simulink model "AcasXuClosedLoop_all_comp2017b"  (Simulink 9.0)
settings: Solver=VariableStepAuto  StartTime=0.0  StopTime=20  RelTol=1e-3
libraries: neural x14040, simulink x2
(part 11 of 534)

== system AcasXuClosedLoop_all_comp2017b/networks/Networks_m_2/AcasXu_2_2/Process Input 1 ==
  x : Inport  PortDimensions=5
      mask Display: e = 0.06;c1 = [120 230 180]/255;c2 = ([120 230 180]+0)/(256+128);c3 = ([120 230 180]+128)/(256+128);patch([0 1 1 0],[0 0 1 1],c1);patch([0 1 1 1-e 1-e 0+e],[0 0 1 1-e 0+e 0+e],c2);patch([0 0 1 1-e 0+e 0+e],[0 1 1 1-e 1-e 0+e],c3);plot([0 1 1 0 0],[0 0 1 1 0]);
  p : Outport  PortDimensions=5
      mask Display: e = 0.06;c1 = [120 230 180]/255;c2 = ([120 230 180]+0)/(256+128);c3 = ([120 230 180]+128)/(256+128);patch([0 1 1 0],[0 0 1 1],c1);patch([0 1 1 1-e 1-e 0+e],[0 0 1 1-e 0+e 0+e],c2);patch([0 0 1 1-e 0+e 0+e],[0 1 1 1-e 1-e 0+e],c3);plot([0 1 1 0 0],[0 0 1 1 0]);
  wires:
  x/1 -> p/1

== system AcasXuClosedLoop_all_comp2017b/networks/Networks_m_2/AcasXu_2_2/Process Output 1 ==
  a : Inport  PortDimensions=5
      mask Display: e = 0.06;c1 = [120 230 180]/255;c2 = ([120 230 180]+0)/(256+128);c3 = ([120 230 180]+128)/(256+128);patch([0 1 1 0],[0 0 1 1],c1);patch([0 1 1 1-e 1-e 0+e],[0 0 1 1-e 0+e 0+e],c2);patch([0 0 1 1-e 0+e 0+e],[0 1 1 1-e 1-e 0+e],c3);plot([0 1 1 0 0],[0 0 1 1 0]);
  y : Outport  PortDimensions=5
      mask Display: e = 0.06;c1 = [120 230 180]/255;c2 = ([120 230 180]+0)/(256+128);c3 = ([120 230 180]+128)/(256+128);patch([0 1 1 0],[0 0 1 1],c1);patch([0 1 1 1-e 1-e 0+e],[0 0 1 1-e 0+e 0+e],c2);patch([0 0 1 1-e 0+e 0+e],[0 1 1 1-e 1-e 0+e],c3);plot([0 1 1 0 0],[0 0 1 1 0]);
  wires:
  a/1 -> y/1

== system AcasXuClosedLoop_all_comp2017b/networks/Networks_m_2/AcasXu_3_2/Layer 1 ==
  "p{1}" : Inport  PortDimensions=5
      mask Display: e = 0.06;c1 = [120 230 180]/255;c2 = ([120 230 180]+0)/(256+128);c3 = ([120 230 180]+128)/(256+128);patch([0 1 1 0],[0 0 1 1],c1);patch([0 1 1 1-e 1-e 0+e],[0 0 1 1-e 0+e 0+e],c2);patch([0 0 1 1-e 0+e 0+e],[0 1 1 1-e 1-e 0+e],c3);plot([0 1 1 0 0],[0 0 1 1 0]);
  Delays 1 : Subsystem
      mask Display: e = 0.06;c1 = [120 150 230]/255;c2 = ([120 150 230]+0)/383;c3 = ([120 150 230]+128)/383;patch([0 1 1 0],[0 0 1 1],c1);patch([0 1 1 1-e 1-e 0+e],[0 0 1 1-e 0+e 0+e],c2);patch([0 0 1 1-e 0+e 0+e],[0 1 1 1-e 1-e 0+e],c3);plot([0 1 1 0 0],[0 0 1 1 0]);disp('0');
  "IW{1,1}" : Subsystem
      mask Display: e = 0.06;c1 = [120 150 230]/255;c2 = ([120 150 230]+0)/383;c3 = ([120 150 230]+128)/383;patch([0 1 1 0],[0 0 1 1],c1);patch([0 1 1 1-e 1-e 0+e],[0 0 1 1-e 0+e 0+e],c2);patch([0 0 1 1-e 0+e 0+e],[0 1 1 1-e 1-e 0+e],c3);plot([0 1 1 0 0],[0 0 1 1 0]);disp('W');
  "b{1}" : Constant  Value=[0.0283022999999999989861887428332920535467565059661865234375;-0.301012000000000001787014980436651967465877532958984375;0.11993800000000000294253510446651489473879337310791015625;0.2316000000000000003108624468950438313186168670654296875;-0.11227900000000000380051545789683586917817592620849609375;-0.417827000000000003954170324504957534372806549072265625;-0.65632100000000004325073632571729831397533416748046875;-0.200220000000000009077183449335279874503612518310546875;-0.388517999999999974480857645176001824438571929931640625;-0.47899300000000000210320649784989655017852783203125;-0.0209766000000000014946710535923557472415268421173095703125;-0.00976452000000000049084736275517570902593433856964111328125;-0.017845300000000001439115493440112913958728313446044921875;-0.1511429999999999995718980017045396380126476287841796875;0.10026500000000000689670542897147242911159992218017578125;-0.28527299999999999879918277656543068587779998779296875;-0.3036659999999999914876980255939997732639312744140625;-0.196284999999999987263521461500204168260097503662109375;-0.111543000000000003257838443460059352219104766845703125;-0.69079800000000002313527147634886205196380615234375;-0.021684599999999998320987515398883260786533355712890625;-0.538942000000000032144953365786932408809661865234375;0.240161000000000013354650718611082993447780609130859375;-0.469312000000000006938449814697378315031528472900390625;-0.44349899999999997657340600198949687182903289794921875;-0.661278999999999950176743368501774966716766357421875;-0.012396600000000000674216238394365063868463039398193359375;-0.1601599999999999968114394732765504159033298492431640625;-0.259077999999999974978237560208071954548358917236328125;0.0317875999999999991896260098656057380139827728271484375;0.0017260999999999999433841768592401422210969030857086181640625;-0.295569000000000026151525389650487340986728668212890625;0.151245999999999991558752299170009791851043701171875;0.07170700000000000684519108062886516563594341278076171875;0.01310560000000000023534507676004068343900144100189208984375;0.12482000000000000039523939676655572839081287384033203125;-0.486669999999999991491250739272800274193286895751953125;0.1386159999999999892228430553586804307997226715087890625;-0.4859510000000000218278728425502777099609375;-0.1790609999999999979447551368139102123677730560302734375;0.461324999999999985078602549037896096706390380859375;-0.01060919999999999918660620323862531222403049468994140625;0.45652700000000001612221467439667321741580963134765625;0.09737079999999999346815826584133901633322238922119140625;0.17900199999999999445066123371361754834651947021484375;-0.856346999999999969332975524594075977802276611328125;-0.52729499999999995818456000051810406148433685302734375;-0.69184900000000004727240821011946536600589752197265625;-0.194915000000000004920508445138693787157535552978515625;-0.642387999999999959044316710787825286388397216796875]
      mask Display: e = 0.06;c1 = [120 150 230]/255;c2 = ([120 150 230]+0)/383;c3 = ([120 150 230]+128)/383;patch([0 1 1 0],[0 0 1 1],c1);patch([0 1 1 1-e 1-e 0+e],[0 0 1 1-e 0+e 0+e],c2);patch([0 0 1 1-e 0+e 0+e],[0 1 1 1-e 1-e 0+e],c3);plot([0 1 1 0 0],[0 0 1 1 0]);disp('b');
  netsum : Sum  Inputs=2  SaturateOnIntegerOverflow=on  InputSameDT=on  OutDataTypeStr=Inherit: Same as first input
      mask Display: e = 0.06;c1 = [120 150 230]/255;c2 = ([120 150 230]+0)/383;c3 = ([120 150 230]+128)/383;patch([0 1 1 0],[0 0 1 1],c1);patch([0 1 1 1-e 1-e 0+e],[0 0 1 1-e 0+e 0+e],c2);patch([0 0 1 1-e 0+e 0+e],[0 1 1 1-e 1-e 0+e],c3);plot([0 1 1 0 0],[0 0 1 1 0]);disp('+');
  poslin : neural/Transfer Functions/poslin
      settings: SourceProductName=Deep Learning Toolbox  SourceProductBaseCode=NN
  "a{1}" : Outport  InitialOutput=[0;0;0;0;0;0;0;0;0;0;0;0;0;0;0;0;0;0;0;0;0;0;0;0;0;0;0;0;0;0;0;0;0;0;0;0;0;0;0;0;0;0;0;0;0;0;0;0;0;0]
      mask Display: e = 0.06;c1 = [120 230 180]/255;c2 = ([120 230 180]+0)/(256+128);c3 = ([120 230 180]+128)/(256+128);patch([0 1 1 0],[0 0 1 1],c1);patch([0 1 1 1-e 1-e 0+e],[0 0 1 1-e 0+e 0+e],c2);patch([0 0 1 1-e 0+e 0+e],[0 1 1 1-e 1-e 0+e],c3);plot([0 1 1 0 0],[0 0 1 1 0]);
  wires:
  poslin/1 -> "a{1}"/1
  netsum/1 -> poslin/1
  "b{1}"/1 -> netsum/2
  "IW{1,1}"/1("iz{1,1}") -> netsum/1
  Delays 1/1("pd{1,1}") -> "IW{1,1}"/1("pd{1,1}")
  "p{1}"/1 -> Delays 1/1("p{1}")

== system AcasXuClosedLoop_all_comp2017b/networks/Networks_m_2/AcasXu_3_2/Layer 2 ==
  "a{1} " : Inport  PortDimensions=50
      mask Display: e = 0.06;c1 = [120 230 180]/255;c2 = ([120 230 180]+0)/(256+128);c3 = ([120 230 180]+128)/(256+128);patch([0 1 1 0],[0 0 1 1],c1);patch([0 1 1 1-e 1-e 0+e],[0 0 1 1-e 0+e 0+e],c2);patch([0 0 1 1-e 0+e 0+e],[0 1 1 1-e 1-e 0+e],c3);plot([0 1 1 0 0],[0 0 1 1 0]);
  Delays 1 : Subsystem
      mask Display: e = 0.06;c1 = [120 150 230]/255;c2 = ([120 150 230]+0)/383;c3 = ([120 150 230]+128)/383;patch([0 1 1 0],[0 0 1 1],c1);patch([0 1 1 1-e 1-e 0+e],[0 0 1 1-e 0+e 0+e],c2);patch([0 0 1 1-e 0+e 0+e],[0 1 1 1-e 1-e 0+e],c3);plot([0 1 1 0 0],[0 0 1 1 0]);disp('0');
  "LW{2,1}" : Subsystem
      mask Display: e = 0.06;c1 = [120 150 230]/255;c2 = ([120 150 230]+0)/383;c3 = ([120 150 230]+128)/383;patch([0 1 1 0],[0 0 1 1],c1);patch([0 1 1 1-e 1-e 0+e],[0 0 1 1-e 0+e 0+e],c2);patch([0 0 1 1-e 0+e 0+e],[0 1 1 1-e 1-e 0+e],c3);plot([0 1 1 0 0],[0 0 1 1 0]);disp('W');
  "b{2}" : Constant  Value=[-0.5780849999999999599964439767063595354557037353515625;0.09880029999999999368309744340876932255923748016357421875;-0.5217330000000000023163693185779266059398651123046875;-0.6774400000000000421351842305739410221576690673828125;-0.0156640000000000008395506512215433758683502674102783203125;1.119639999999999968594011079403571784496307373046875;0.318240999999999996106225808034650981426239013671875;0.5233790000000000386393139706342481076717376708984375;0.34323300000000001030997509587905369699001312255859375;1.44550000000000000710542735760100185871124267578125;0.0244362000000000016697310201152504305355250835418701171875;-0.838797000000000014807710613240487873554229736328125;-0.005467689999999999876656442410194358672015368938446044921875;0.2122489999999999932267513713668449781835079193115234375;-0.53288100000000004907718675895011983811855316162109375;-0.06930350000000000398880928287326241843402385711669921875;-1.100680000000000102744479590910486876964569091796875;-1.01367999999999991445065461448393762111663818359375;-0.195641000000000009340084261566516943275928497314453125;0.07954650000000000609201578072315896861255168914794921875;0.6645280000000000075743855632026679813861846923828125;-0.34353800000000001002575800157501362264156341552734375;-0.01464500000000000003164135620181696140207350254058837890625;0.6232640000000000402025079893064685165882110595703125;0.68372500000000002717825964282383210957050323486328125;-0.0195708999999999987140508750371736823581159114837646484375;-0.09863909999999999345110524018309661187231540679931640625;0.442062000000000010491163493497879244387149810791015625;-0.2537409999999999943298689686344005167484283447265625;0.442377999999999993452348689970676787197589874267578125;-0.11313099999999999545341466955505893565714359283447265625;-0.51815500000000003222311306672054342925548553466796875;-0.0237068999999999995009769548914846382103860378265380859375;0.2077400000000000079847239931041258387267589569091796875;-0.69884199999999996322230799705721437931060791015625;0.121782000000000001360689338980591855943202972412109375;-0.9777430000000000287485590888536535203456878662109375;-1.0094300000000000494537744089029729366302490234375;-0.692020999999999997243094185250811278820037841796875;0.9666550000000000419930756834219209849834442138671875;-0.946938999999999975187847667257301509380340576171875;0.207311999999999996280308778295875526964664459228515625;-0.1005540000000000044888537331644329242408275604248046875;-0.271942999999999990290433515838230960071086883544921875;0.57471499999999997587707412094459868967533111572265625;-0.48166799999999998505018083960749208927154541015625;0.04291760000000000019326762412674725055694580078125;0.48387299999999999755573298898525536060333251953125;-0.026528099999999998903632558722165413200855255126953125;-0.29513800000000001144684347309521399438381195068359375]
      mask Display: e = 0.06;c1 = [120 150 230]/255;c2 = ([120 150 230]+0)/383;c3 = ([120 150 230]+128)/383;patch([0 1 1 0],[0 0 1 1],c1);patch([0 1 1 1-e 1-e 0+e],[0 0 1 1-e 0+e 0+e],c2);patch([0 0 1 1-e 0+e 0+e],[0 1 1 1-e 1-e 0+e],c3);plot([0 1 1 0 0],[0 0 1 1 0]);disp('b');
  netsum : Sum  Inputs=2  SaturateOnIntegerOverflow=on  InputSameDT=on  OutDataTypeStr=Inherit: Same as first input
      mask Display: e = 0.06;c1 = [120 150 230]/255;c2 = ([120 150 230]+0)/383;c3 = ([120 150 230]+128)/383;patch([0 1 1 0],[0 0 1 1],c1);patch([0 1 1 1-e 1-e 0+e],[0 0 1 1-e 0+e 0+e],c2);patch([0 0 1 1-e 0+e 0+e],[0 1 1 1-e 1-e 0+e],c3);plot([0 1 1 0 0],[0 0 1 1 0]);disp('+');
  poslin : neural/Transfer Functions/poslin
      settings: SourceProductName=Deep Learning Toolbox  SourceProductBaseCode=NN
  "a{2}" : Outport  InitialOutput=[0;0;0;0;0;0;0;0;0;0;0;0;0;0;0;0;0;0;0;0;0;0;0;0;0;0;0;0;0;0;0;0;0;0;0;0;0;0;0;0;0;0;0;0;0;0;0;0;0;0]
      mask Display: e = 0.06;c1 = [120 230 180]/255;c2 = ([120 230 180]+0)/(256+128);c3 = ([120 230 180]+128)/(256+128);patch([0 1 1 0],[0 0 1 1],c1);patch([0 1 1 1-e 1-e 0+e],[0 0 1 1-e 0+e 0+e],c2);patch([0 0 1 1-e 0+e 0+e],[0 1 1 1-e 1-e 0+e],c3);plot([0 1 1 0 0],[0 0 1 1 0]);
  wires:
  poslin/1 -> "a{2}"/1
  netsum/1 -> poslin/1
  "b{2}"/1 -> netsum/2
  "LW{2,1}"/1("lz{2,1}") -> netsum/1
  Delays 1/1("pd{2,1}") -> "LW{2,1}"/1("ad{2,1}")
  "a{1} "/1 -> Delays 1/1("p{2}")

== system AcasXuClosedLoop_all_comp2017b/networks/Networks_m_2/AcasXu_3_2/Layer 3 ==
  "a{2} " : Inport  PortDimensions=50
      mask Display: e = 0.06;c1 = [120 230 180]/255;c2 = ([120 230 180]+0)/(256+128);c3 = ([120 230 180]+128)/(256+128);patch([0 1 1 0],[0 0 1 1],c1);patch([0 1 1 1-e 1-e 0+e],[0 0 1 1-e 0+e 0+e],c2);patch([0 0 1 1-e 0+e 0+e],[0 1 1 1-e 1-e 0+e],c3);plot([0 1 1 0 0],[0 0 1 1 0]);
  Delays 1 : Subsystem
      mask Display: e = 0.06;c1 = [120 150 230]/255;c2 = ([120 150 230]+0)/383;c3 = ([120 150 230]+128)/383;patch([0 1 1 0],[0 0 1 1],c1);patch([0 1 1 1-e 1-e 0+e],[0 0 1 1-e 0+e 0+e],c2);patch([0 0 1 1-e 0+e 0+e],[0 1 1 1-e 1-e 0+e],c3);plot([0 1 1 0 0],[0 0 1 1 0]);disp('0');
  "LW{3,2}" : Subsystem
      mask Display: e = 0.06;c1 = [120 150 230]/255;c2 = ([120 150 230]+0)/383;c3 = ([120 150 230]+128)/383;patch([0 1 1 0],[0 0 1 1],c1);patch([0 1 1 1-e 1-e 0+e],[0 0 1 1-e 0+e 0+e],c2);patch([0 0 1 1-e 0+e 0+e],[0 1 1 1-e 1-e 0+e],c3);plot([0 1 1 0 0],[0 0 1 1 0]);disp('W');
  "b{3}" : Constant  Value=[0.23227100000000000523669996255193836987018585205078125;0.5344139999999999446345100295729935169219970703125;-0.032794999999999997764010828404934727586805820465087890625;-0.2236120000000000052065018962821341119706630706787109375;-1.03896999999999994912514011957682669162750244140625;-0.69521800000000000263611354966997168958187103271484375;0.22183100000000000040500935938325710594654083251953125;-0.1544129999999999947046802617478533647954463958740234375;-0.427362000000000019639401216409169137477874755859375;0.005143899999999999861355348684810451231896877288818359375;0.898020999999999958163243718445301055908203125;-0.324894999999999989359622531992499716579914093017578125;-0.1422279999999999933191219270156580023467540740966796875;1.2399100000000000676436684443615376949310302734375;-0.06305249999999999743760525916513870470225811004638671875;-0.0772072000000000036035174844073480926454067230224609375;1.4434199999999999253219584716134704649448394775390625;-1.875189999999999912461134954355657100677490234375;0.2173950000000000049027448767446912825107574462890625;0.10771999999999999630961866614597965963184833526611328125;0.367703999999999975312192646015319041907787322998046875;-0.0146190999999999994563015803805683390237390995025634765625;-0.84726500000000004586553359331446699798107147216796875;-0.77965099999999998292565805968479253351688385009765625;-0.359457999999999999740651901447563432157039642333984375;0.9602500000000000479616346638067625463008880615234375;-0.033392600000000001447819641953174141235649585723876953125;0.27712199999999997945820950917550362646579742431640625;-0.6815780000000000171667124959640204906463623046875;-0.15326600000000001333688715021708048880100250244140625;2.0688300000000001688249540165998041629791259765625;2.21379999999999999005240169935859739780426025390625;-0.9270110000000000294306801151833496987819671630859375;-0.1576019999999999920969884215082856826484203338623046875;0.294466999999999978765430341809405945241451263427734375;-1.5096499999999999364064251494710333645343780517578125;-0.9974840000000000372892827726900577545166015625;-1.299139999999999961488583721802569925785064697265625;0.05077199999999999768807157352057402022182941436767578125;0.01490380000000000003390621117205228074453771114349365234375;0.17562400000000000233058017329312860965728759765625;-0.356165000000000009361400543639319948852062225341796875;-0.298904000000000003023359340659226290881633758544921875;0.2238030000000000019344525981068727560341358184814453125;0.94540699999999999736388645033002831041812896728515625;0.59672300000000000341771055900608189404010772705078125;0.265276000000000011791456699938862584531307220458984375;0.059614599999999996760013942775913164950907230377197265625;0.48969099999999998740207729497342370450496673583984375;-0.59394000000000002348343741687131114304065704345703125]
      mask Display: e = 0.06;c1 = [120 150 230]/255;c2 = ([120 150 230]+0)/383;c3 = ([120 150 230]+128)/383;patch([0 1 1 0],[0 0 1 1],c1);patch([0 1 1 1-e 1-e 0+e],[0 0 1 1-e 0+e 0+e],c2);patch([0 0 1 1-e 0+e 0+e],[0 1 1 1-e 1-e 0+e],c3);plot([0 1 1 0 0],[0 0 1 1 0]);disp('b');
  netsum : Sum  Inputs=2  SaturateOnIntegerOverflow=on  InputSameDT=on  OutDataTypeStr=Inherit: Same as first input
      mask Display: e = 0.06;c1 = [120 150 230]/255;c2 = ([120 150 230]+0)/383;c3 = ([120 150 230]+128)/383;patch([0 1 1 0],[0 0 1 1],c1);patch([0 1 1 1-e 1-e 0+e],[0 0 1 1-e 0+e 0+e],c2);patch([0 0 1 1-e 0+e 0+e],[0 1 1 1-e 1-e 0+e],c3);plot([0 1 1 0 0],[0 0 1 1 0]);disp('+');
  poslin : neural/Transfer Functions/poslin
      settings: SourceProductName=Deep Learning Toolbox  SourceProductBaseCode=NN
  "a{3}" : Outport  InitialOutput=[0;0;0;0;0;0;0;0;0;0;0;0;0;0;0;0;0;0;0;0;0;0;0;0;0;0;0;0;0;0;0;0;0;0;0;0;0;0;0;0;0;0;0;0;0;0;0;0;0;0]
      mask Display: e = 0.06;c1 = [120 230 180]/255;c2 = ([120 230 180]+0)/(256+128);c3 = ([120 230 180]+128)/(256+128);patch([0 1 1 0],[0 0 1 1],c1);patch([0 1 1 1-e 1-e 0+e],[0 0 1 1-e 0+e 0+e],c2);patch([0 0 1 1-e 0+e 0+e],[0 1 1 1-e 1-e 0+e],c3);plot([0 1 1 0 0],[0 0 1 1 0]);
  wires:
  poslin/1 -> "a{3}"/1
  netsum/1 -> poslin/1
  "b{3}"/1 -> netsum/2
  "LW{3,2}"/1("lz{3,2}") -> netsum/1
  Delays 1/1("pd{3,2}") -> "LW{3,2}"/1("ad{3,2}")
  "a{2} "/1 -> Delays 1/1("p{3}")

== system AcasXuClosedLoop_all_comp2017b/networks/Networks_m_2/AcasXu_3_2/Layer 4 ==
  "a{3} " : Inport  PortDimensions=50
      mask Display: e = 0.06;c1 = [120 230 180]/255;c2 = ([120 230 180]+0)/(256+128);c3 = ([120 230 180]+128)/(256+128);patch([0 1 1 0],[0 0 1 1],c1);patch([0 1 1 1-e 1-e 0+e],[0 0 1 1-e 0+e 0+e],c2);patch([0 0 1 1-e 0+e 0+e],[0 1 1 1-e 1-e 0+e],c3);plot([0 1 1 0 0],[0 0 1 1 0]);
  Delays 1 : Subsystem
      mask Display: e = 0.06;c1 = [120 150 230]/255;c2 = ([120 150 230]+0)/383;c3 = ([120 150 230]+128)/383;patch([0 1 1 0],[0 0 1 1],c1);patch([0 1 1 1-e 1-e 0+e],[0 0 1 1-e 0+e 0+e],c2);patch([0 0 1 1-e 0+e 0+e],[0 1 1 1-e 1-e 0+e],c3);plot([0 1 1 0 0],[0 0 1 1 0]);disp('0');
  "LW{4,3}" : Subsystem
      mask Display: e = 0.06;c1 = [120 150 230]/255;c2 = ([120 150 230]+0)/383;c3 = ([120 150 230]+128)/383;patch([0 1 1 0],[0 0 1 1],c1);patch([0 1 1 1-e 1-e 0+e],[0 0 1 1-e 0+e 0+e],c2);patch([0 0 1 1-e 0+e 0+e],[0 1 1 1-e 1-e 0+e],c3);plot([0 1 1 0 0],[0 0 1 1 0]);disp('W');
  "b{4}" : Constant  Value=[-0.6134100000000000108002495835535228252410888671875;-0.202566999999999997061905787631985731422901153564453125;0.204064999999999996393995616017491556704044342041015625;0.44556899999999999284483465089579112827777862548828125;-0.48840899999999998204458506734226830303668975830078125;0.5372879999999999878212975090718828141689300537109375;-1.02038999999999990819787853979505598545074462890625;0.1576390000000000013447021274259896017611026763916015625;-0.63987600000000000033395508580724708735942840576171875;0.72517299999999995652188999883946962654590606689453125;-0.20397100000000001340794142379309050738811492919921875;-0.2003020000000000078177464502005022950470447540283203125;-0.41732000000000002426503442620742134749889373779296875;0.9708040000000000002700062395888380706310272216796875;-0.058541000000000002756461725539338658563792705535888671875;0.28557100000000001926991899381391704082489013671875;-0.46571899999999999408828443847596645355224609375;0.1843950000000000033484326422694721259176731109619140625;-0.10219699999999999617816826003036112524569034576416015625;0.8542359999999999953246287986985407769680023193359375;-0.2026820000000000010498268920855480246245861053466796875;-1.5198199999999999487698687516967765986919403076171875;-1.3590199999999998947686208339291624724864959716796875;-0.6151839999999999530899685851181857287883758544921875;-1.5441499999999999115374293978675268590450286865234375;0.51265499999999997182698052711202763020992279052734375;-0.178914999999999990709653729936690069735050201416015625;0.236866999999999994219734844591584987938404083251953125;-1.79445999999999994400923242210410535335540771484375;-1.4061600000000000765254526413627900183200836181640625;-0.34094400000000002481925776010029949247837066650390625;-0.0211470999999999985374810052007887861691415309906005859375;-0.60485800000000000675726141707855276763439178466796875;0.1150110000000000021191937094044988043606281280517578125;-1.29746000000000005769607014372013509273529052734375;0.440852999999999994873434161490877158939838409423828125;-0.0218700999999999999567901198815889074467122554779052734375;1.424679999999999946425077723688445985317230224609375;0.1911380000000000023430146711689303629100322723388671875;-0.046478100000000001357225443143761367537081241607666015625;0.21815499999999998781419208171428181231021881103515625;-0.09141870000000000551221290834291721694171428680419921875;-0.0785238000000000047062798103070235811173915863037109375;-0.0212777000000000000856648085800770786590874195098876953125;-0.013587200000000000665867361249183886684477329254150390625;0.048669099999999999861532984368750476278364658355712890625;-1.9603200000000000624567064733128063380718231201171875;0.262436999999999975852205125192995183169841766357421875;0.51545300000000005002931402486865408718585968017578125;-3.0631099999999999994315658113919198513031005859375]
      mask Display: e = 0.06;c1 = [120 150 230]/255;c2 = ([120 150 230]+0)/383;c3 = ([120 150 230]+128)/383;patch([0 1 1 0],[0 0 1 1],c1);patch([0 1 1 1-e 1-e 0+e],[0 0 1 1-e 0+e 0+e],c2);patch([0 0 1 1-e 0+e 0+e],[0 1 1 1-e 1-e 0+e],c3);plot([0 1 1 0 0],[0 0 1 1 0]);disp('b');
  netsum : Sum  Inputs=2  SaturateOnIntegerOverflow=on  InputSameDT=on  OutDataTypeStr=Inherit: Same as first input
      mask Display: e = 0.06;c1 = [120 150 230]/255;c2 = ([120 150 230]+0)/383;c3 = ([120 150 230]+128)/383;patch([0 1 1 0],[0 0 1 1],c1);patch([0 1 1 1-e 1-e 0+e],[0 0 1 1-e 0+e 0+e],c2);patch([0 0 1 1-e 0+e 0+e],[0 1 1 1-e 1-e 0+e],c3);plot([0 1 1 0 0],[0 0 1 1 0]);disp('+');
  poslin : neural/Transfer Functions/poslin
      settings: SourceProductName=Deep Learning Toolbox  SourceProductBaseCode=NN
  "a{4}" : Outport  InitialOutput=[0;0;0;0;0;0;0;0;0;0;0;0;0;0;0;0;0;0;0;0;0;0;0;0;0;0;0;0;0;0;0;0;0;0;0;0;0;0;0;0;0;0;0;0;0;0;0;0;0;0]
      mask Display: e = 0.06;c1 = [120 230 180]/255;c2 = ([120 230 180]+0)/(256+128);c3 = ([120 230 180]+128)/(256+128);patch([0 1 1 0],[0 0 1 1],c1);patch([0 1 1 1-e 1-e 0+e],[0 0 1 1-e 0+e 0+e],c2);patch([0 0 1 1-e 0+e 0+e],[0 1 1 1-e 1-e 0+e],c3);plot([0 1 1 0 0],[0 0 1 1 0]);
  wires:
  poslin/1 -> "a{4}"/1
  netsum/1 -> poslin/1
  "b{4}"/1 -> netsum/2
  "LW{4,3}"/1("lz{4,3}") -> netsum/1
  Delays 1/1("pd{4,3}") -> "LW{4,3}"/1("ad{4,3}")
  "a{3} "/1 -> Delays 1/1("p{4}")

== system AcasXuClosedLoop_all_comp2017b/networks/Networks_m_2/AcasXu_3_2/Layer 5 ==
  "a{4} " : Inport  PortDimensions=50
      mask Display: e = 0.06;c1 = [120 230 180]/255;c2 = ([120 230 180]+0)/(256+128);c3 = ([120 230 180]+128)/(256+128);patch([0 1 1 0],[0 0 1 1],c1);patch([0 1 1 1-e 1-e 0+e],[0 0 1 1-e 0+e 0+e],c2);patch([0 0 1 1-e 0+e 0+e],[0 1 1 1-e 1-e 0+e],c3);plot([0 1 1 0 0],[0 0 1 1 0]);
  Delays 1 : Subsystem
      mask Display: e = 0.06;c1 = [120 150 230]/255;c2 = ([120 150 230]+0)/383;c3 = ([120 150 230]+128)/383;patch([0 1 1 0],[0 0 1 1],c1);patch([0 1 1 1-e 1-e 0+e],[0 0 1 1-e 0+e 0+e],c2);patch([0 0 1 1-e 0+e 0+e],[0 1 1 1-e 1-e 0+e],c3);plot([0 1 1 0 0],[0 0 1 1 0]);disp('0');
  "LW{5,4}" : Subsystem
      mask Display: e = 0.06;c1 = [120 150 230]/255;c2 = ([120 150 230]+0)/383;c3 = ([120 150 230]+128)/383;patch([0 1 1 0],[0 0 1 1],c1);patch([0 1 1 1-e 1-e 0+e],[0 0 1 1-e 0+e 0+e],c2);patch([0 0 1 1-e 0+e 0+e],[0 1 1 1-e 1-e 0+e],c3);plot([0 1 1 0 0],[0 0 1 1 0]);disp('W');
  "b{5}" : Constant  Value=[-0.255139999999999977919884486254886724054813385009765625;-1.134139999999999925961446933797560632228851318359375;-0.030468900000000000150013335087351151742041110992431640625;0.0892741999999999980008880129389581270515918731689453125;-0.0287665000000000004032330025438568554818630218505859375;-1.27062000000000008270717444247566163539886474609375;0.471642999999999978921749743676627986133098602294921875;-0.46033299999999999219113533399649895727634429931640625;-0.019451099999999998946265122867771424353122711181640625;-0.7328799999999999759126012577326036989688873291015625;-0.680525999999999964273911245982162654399871826171875;-0.6588110000000000354702933691442012786865234375;-0.2075460000000000082565065895323641598224639892578125;-2.525440000000000129176669361186213791370391845703125;-0.1319909999999999972164488326598075218498706817626953125;0.1363350000000000117328369242386543191969394683837890625;0.6334020000000000205631067728972993791103363037109375;-0.11449199999999999655120319630441372282803058624267578125;0.415024000000000004018119170723366551101207733154296875;0.74063800000000001855227083069621585309505462646484375;-0.172038999999999997481126001730444841086864471435546875;-0.89461599999999996679633795793051831424236297607421875;-0.358445999999999986851406674759346060454845428466796875;-0.705594000000000054484416978084482252597808837890625;-0.356157000000000001360689338980591855943202972412109375;-1.834140000000000103597130873822607100009918212890625;-0.173937000000000008270717444247566163539886474609375;0.381763000000000018996360040546278469264507293701171875;-1.01811000000000007048583938740193843841552734375;0.1160930000000000017479351299698464572429656982421875;0.611253000000000046298964662128128111362457275390625;0.953014000000000027768010113504715263843536376953125;0.16672799999999998732391759403981268405914306640625;1.585970000000000101891828307998366653919219970703125;-1.6443600000000000438404867963981814682483673095703125;-2.447270000000000056417093219351954758167266845703125;-0.8077229999999999687787521907011978328227996826171875;-0.35090500000000002245315045001916587352752685546875;-0.0173653999999999995862420831826966605149209499359130859375;0.125607999999999997431388010227237828075885772705078125;-0.721848000000000045162096284911967813968658447265625;-0.7378240000000000353708173861377872526645660400390625;0.59798099999999998477306917266105301678180694580078125;0.69380299999999994753352439147420227527618408203125;0.429259000000000001673328142715035937726497650146484375;-0.49782300000000001549693706692778505384922027587890625;0.43925300000000000455457893622224219143390655517578125;-0.93269100000000004779820983458193950355052947998046875;-0.0595878999999999992009946936377673409879207611083984375;-0.72655499999999995086596982218907214701175689697265625]
      mask Display: e = 0.06;c1 = [120 150 230]/255;c2 = ([120 150 230]+0)/383;c3 = ([120 150 230]+128)/383;patch([0 1 1 0],[0 0 1 1],c1);patch([0 1 1 1-e 1-e 0+e],[0 0 1 1-e 0+e 0+e],c2);patch([0 0 1 1-e 0+e 0+e],[0 1 1 1-e 1-e 0+e],c3);plot([0 1 1 0 0],[0 0 1 1 0]);disp('b');
  netsum : Sum  Inputs=2  SaturateOnIntegerOverflow=on  InputSameDT=on  OutDataTypeStr=Inherit: Same as first input
      mask Display: e = 0.06;c1 = [120 150 230]/255;c2 = ([120 150 230]+0)/383;c3 = ([120 150 230]+128)/383;patch([0 1 1 0],[0 0 1 1],c1);patch([0 1 1 1-e 1-e 0+e],[0 0 1 1-e 0+e 0+e],c2);patch([0 0 1 1-e 0+e 0+e],[0 1 1 1-e 1-e 0+e],c3);plot([0 1 1 0 0],[0 0 1 1 0]);disp('+');
  poslin : neural/Transfer Functions/poslin
      settings: SourceProductName=Deep Learning Toolbox  SourceProductBaseCode=NN
  "a{5}" : Outport  InitialOutput=[0;0;0;0;0;0;0;0;0;0;0;0;0;0;0;0;0;0;0;0;0;0;0;0;0;0;0;0;0;0;0;0;0;0;0;0;0;0;0;0;0;0;0;0;0;0;0;0;0;0]
      mask Display: e = 0.06;c1 = [120 230 180]/255;c2 = ([120 230 180]+0)/(256+128);c3 = ([120 230 180]+128)/(256+128);patch([0 1 1 0],[0 0 1 1],c1);patch([0 1 1 1-e 1-e 0+e],[0 0 1 1-e 0+e 0+e],c2);patch([0 0 1 1-e 0+e 0+e],[0 1 1 1-e 1-e 0+e],c3);plot([0 1 1 0 0],[0 0 1 1 0]);
  wires:
  poslin/1 -> "a{5}"/1
  netsum/1 -> poslin/1
  "b{5}"/1 -> netsum/2
  "LW{5,4}"/1("lz{5,4}") -> netsum/1
  Delays 1/1("pd{5,4}") -> "LW{5,4}"/1("ad{5,4}")
  "a{4} "/1 -> Delays 1/1("p{5}")

== system AcasXuClosedLoop_all_comp2017b/networks/Networks_m_2/AcasXu_3_2/Layer 6 ==
  "a{5} " : Inport  PortDimensions=50
      mask Display: e = 0.06;c1 = [120 230 180]/255;c2 = ([120 230 180]+0)/(256+128);c3 = ([120 230 180]+128)/(256+128);patch([0 1 1 0],[0 0 1 1],c1);patch([0 1 1 1-e 1-e 0+e],[0 0 1 1-e 0+e 0+e],c2);patch([0 0 1 1-e 0+e 0+e],[0 1 1 1-e 1-e 0+e],c3);plot([0 1 1 0 0],[0 0 1 1 0]);
  Delays 1 : Subsystem
      mask Display: e = 0.06;c1 = [120 150 230]/255;c2 = ([120 150 230]+0)/383;c3 = ([120 150 230]+128)/383;patch([0 1 1 0],[0 0 1 1],c1);patch([0 1 1 1-e 1-e 0+e],[0 0 1 1-e 0+e 0+e],c2);patch([0 0 1 1-e 0+e 0+e],[0 1 1 1-e 1-e 0+e],c3);plot([0 1 1 0 0],[0 0 1 1 0]);disp('0');
  "LW{6,5}" : Subsystem
      mask Display: e = 0.06;c1 = [120 150 230]/255;c2 = ([120 150 230]+0)/383;c3 = ([120 150 230]+128)/383;patch([0 1 1 0],[0 0 1 1],c1);patch([0 1 1 1-e 1-e 0+e],[0 0 1 1-e 0+e 0+e],c2);patch([0 0 1 1-e 0+e 0+e],[0 1 1 1-e 1-e 0+e],c3);plot([0 1 1 0 0],[0 0 1 1 0]);disp('W');
  "b{6}" : Constant  Value=[0.462285000000000001474376176702207885682582855224609375;0.251578000000000023828050643714959733188152313232421875;-1.17494999999999993889332472463138401508331298828125;0.1909160000000000023678836669205338694155216217041015625;0.246979000000000004089173444299376569688320159912109375;0.47652499999999997637445403597666881978511810302734375;0.357725999999999988432364261825568974018096923828125;0.7382239999999999913171677690115757286548614501953125;0.25876500000000002277289468111121095716953277587890625;0.205230999999999996763477838612743653357028961181640625;0.51042299999999996007460367763997055590152740478515625;0.1975260000000000071285199965132051147520542144775390625;1.0505299999999999638333747498109005391597747802734375;0.33083899999999999419486584883998148143291473388671875;0.229657000000000000028421709430404007434844970703125;0.689759000000000010999201549566350877285003662109375;0.49633599999999999941024952931911684572696685791015625;-0.161445000000000005169198402654728852212429046630859375;-0.041119099999999998706901038758587674237787723541259765625;-0.9594359999999999555342355961329303681850433349609375;0.375396000000000007457146011802251450717449188232421875;0.082022399999999995312549572190619073808193206787109375;-0.71242199999999999970867747833835892379283905029296875;0.252842999999999984428455945817404426634311676025390625;0.5330209999999999670450279154465533792972564697265625;-1.5556499999999999772626324556767940521240234375;0.61635399999999995745980641004280187189579010009765625;-1.6176999999999999158006858124281279742717742919921875;0.280857999999999996543209590527112595736980438232421875;0.00289731000000000016303403072015498764812946319580078125;0.00250298000000000013531842313341257977299392223358154296875;-0.86735799999999996234834043207229115068912506103515625;0.09032329999999999525783778153709135949611663818359375;0.27224999999999999200639422269887290894985198974609375;0.19258900000000001018207740344223566353321075439453125;0.2059459999999999901820757486348156817257404327392578125;0.283868999999999982453147140404325909912586212158203125;-1.1283600000000000296296320811961777508258819580078125;-0.91287799999999996725108530881698243319988250732421875;0.317446999999999979191755983265466056764125823974609375;-0.28662799999999999389643789982073940336704254150390625;0.44124499999999999833022457096376456320285797119140625;0.0033762900000000001070332711350374665926210582256317138671875;0.1013860000000000038955505488047492690384387969970703125;-1.630030000000000089954710347228683531284332275390625;-0.36274200000000000887467876964365132153034210205078125;0.10867400000000000670485889031624537892639636993408203125;-0.2634659999999999779873860461520962417125701904296875;0.1922089999999999909707781853285268880426883697509765625;0.20384299999999999641886461176909506320953369140625]
      mask Display: e = 0.06;c1 = [120 150 230]/255;c2 = ([120 150 230]+0)/383;c3 = ([120 150 230]+128)/383;patch([0 1 1 0],[0 0 1 1],c1);patch([0 1 1 1-e 1-e 0+e],[0 0 1 1-e 0+e 0+e],c2);patch([0 0 1 1-e 0+e 0+e],[0 1 1 1-e 1-e 0+e],c3);plot([0 1 1 0 0],[0 0 1 1 0]);disp('b');
  netsum : Sum  Inputs=2  SaturateOnIntegerOverflow=on  InputSameDT=on  OutDataTypeStr=Inherit: Same as first input
      mask Display: e = 0.06;c1 = [120 150 230]/255;c2 = ([120 150 230]+0)/383;c3 = ([120 150 230]+128)/383;patch([0 1 1 0],[0 0 1 1],c1);patch([0 1 1 1-e 1-e 0+e],[0 0 1 1-e 0+e 0+e],c2);patch([0 0 1 1-e 0+e 0+e],[0 1 1 1-e 1-e 0+e],c3);plot([0 1 1 0 0],[0 0 1 1 0]);disp('+');
  poslin : neural/Transfer Functions/poslin
      settings: SourceProductName=Deep Learning Toolbox  SourceProductBaseCode=NN
  "a{6}" : Outport  InitialOutput=[0;0;0;0;0;0;0;0;0;0;0;0;0;0;0;0;0;0;0;0;0;0;0;0;0;0;0;0;0;0;0;0;0;0;0;0;0;0;0;0;0;0;0;0;0;0;0;0;0;0]
      mask Display: e = 0.06;c1 = [120 230 180]/255;c2 = ([120 230 180]+0)/(256+128);c3 = ([120 230 180]+128)/(256+128);patch([0 1 1 0],[0 0 1 1],c1);patch([0 1 1 1-e 1-e 0+e],[0 0 1 1-e 0+e 0+e],c2);patch([0 0 1 1-e 0+e 0+e],[0 1 1 1-e 1-e 0+e],c3);plot([0 1 1 0 0],[0 0 1 1 0]);
  wires:
  poslin/1 -> "a{6}"/1
  netsum/1 -> poslin/1
  "b{6}"/1 -> netsum/2
  "LW{6,5}"/1("lz{6,5}") -> netsum/1
  Delays 1/1("pd{6,5}") -> "LW{6,5}"/1("ad{6,5}")
  "a{5} "/1 -> Delays 1/1("p{6}")

== system AcasXuClosedLoop_all_comp2017b/networks/Networks_m_2/AcasXu_3_2/Layer 7 ==
  "a{6} " : Inport  PortDimensions=50
      mask Display: e = 0.06;c1 = [120 230 180]/255;c2 = ([120 230 180]+0)/(256+128);c3 = ([120 230 180]+128)/(256+128);patch([0 1 1 0],[0 0 1 1],c1);patch([0 1 1 1-e 1-e 0+e],[0 0 1 1-e 0+e 0+e],c2);patch([0 0 1 1-e 0+e 0+e],[0 1 1 1-e 1-e 0+e],c3);plot([0 1 1 0 0],[0 0 1 1 0]);
  Delays 1 : Subsystem
      mask Display: e = 0.06;c1 = [120 150 230]/255;c2 = ([120 150 230]+0)/383;c3 = ([120 150 230]+128)/383;patch([0 1 1 0],[0 0 1 1],c1);patch([0 1 1 1-e 1-e 0+e],[0 0 1 1-e 0+e 0+e],c2);patch([0 0 1 1-e 0+e 0+e],[0 1 1 1-e 1-e 0+e],c3);plot([0 1 1 0 0],[0 0 1 1 0]);disp('0');
  "LW{7,6}" : Subsystem
      mask Display: e = 0.06;c1 = [120 150 230]/255;c2 = ([120 150 230]+0)/383;c3 = ([120 150 230]+128)/383;patch([0 1 1 0],[0 0 1 1],c1);patch([0 1 1 1-e 1-e 0+e],[0 0 1 1-e 0+e 0+e],c2);patch([0 0 1 1-e 0+e 0+e],[0 1 1 1-e 1-e 0+e],c3);plot([0 1 1 0 0],[0 0 1 1 0]);disp('W');
  "b{7}" : Constant  Value=[-0.0217394999999999984086063165023006149567663669586181640625;0.017968499999999998362198994072969071567058563232421875;-0.0190985000000000008923972671937008271925151348114013671875;0.0186103999999999991654675568497623316943645477294921875;-0.0180903000000000001301625474070533527992665767669677734375]
      mask Display: e = 0.06;c1 = [120 150 230]/255;c2 = ([120 150 230]+0)/383;c3 = ([120 150 230]+128)/383;patch([0 1 1 0],[0 0 1 1],c1);patch([0 1 1 1-e 1-e 0+e],[0 0 1 1-e 0+e 0+e],c2);patch([0 0 1 1-e 0+e 0+e],[0 1 1 1-e 1-e 0+e],c3);plot([0 1 1 0 0],[0 0 1 1 0]);disp('b');
  netsum : Sum  Inputs=2  SaturateOnIntegerOverflow=on  InputSameDT=on  OutDataTypeStr=Inherit: Same as first input
      mask Display: e = 0.06;c1 = [120 150 230]/255;c2 = ([120 150 230]+0)/383;c3 = ([120 150 230]+128)/383;patch([0 1 1 0],[0 0 1 1],c1);patch([0 1 1 1-e 1-e 0+e],[0 0 1 1-e 0+e 0+e],c2);patch([0 0 1 1-e 0+e 0+e],[0 1 1 1-e 1-e 0+e],c3);plot([0 1 1 0 0],[0 0 1 1 0]);disp('+');
  purelin : neural/Transfer Functions/purelin
      settings: SourceProductName=Deep Learning Toolbox
  "a{7}" : Outport  InitialOutput=[0;0;0;0;0]
      mask Display: e = 0.06;c1 = [120 230 180]/255;c2 = ([120 230 180]+0)/(256+128);c3 = ([120 230 180]+128)/(256+128);patch([0 1 1 0],[0 0 1 1],c1);patch([0 1 1 1-e 1-e 0+e],[0 0 1 1-e 0+e 0+e],c2);patch([0 0 1 1-e 0+e 0+e],[0 1 1 1-e 1-e 0+e],c3);plot([0 1 1 0 0],[0 0 1 1 0]);
  wires:
  purelin/1 -> "a{7}"/1
  netsum/1 -> purelin/1
  "b{7}"/1 -> netsum/2
  "LW{7,6}"/1("lz{7,6}") -> netsum/1
  Delays 1/1("pd{7,6}") -> "LW{7,6}"/1("ad{7,6}")
  "a{6} "/1 -> Delays 1/1("p{7}")

== system AcasXuClosedLoop_all_comp2017b/networks/Networks_m_2/AcasXu_3_2/Process Input 1 ==
  x : Inport  PortDimensions=5
      mask Display: e = 0.06;c1 = [120 230 180]/255;c2 = ([120 230 180]+0)/(256+128);c3 = ([120 230 180]+128)/(256+128);patch([0 1 1 0],[0 0 1 1],c1);patch([0 1 1 1-e 1-e 0+e],[0 0 1 1-e 0+e 0+e],c2);patch([0 0 1 1-e 0+e 0+e],[0 1 1 1-e 1-e 0+e],c3);plot([0 1 1 0 0],[0 0 1 1 0]);
  p : Outport  PortDimensions=5
      mask Display: e = 0.06;c1 = [120 230 180]/255;c2 = ([120 230 180]+0)/(256+128);c3 = ([120 230 180]+128)/(256+128);patch([0 1 1 0],[0 0 1 1],c1);patch([0 1 1 1-e 1-e 0+e],[0 0 1 1-e 0+e 0+e],c2);patch([0 0 1 1-e 0+e 0+e],[0 1 1 1-e 1-e 0+e],c3);plot([0 1 1 0 0],[0 0 1 1 0]);
  wires:
  x/1 -> p/1

== system AcasXuClosedLoop_all_comp2017b/networks/Networks_m_2/AcasXu_3_2/Process Output 1 ==
  a : Inport  PortDimensions=5
      mask Display: e = 0.06;c1 = [120 230 180]/255;c2 = ([120 230 180]+0)/(256+128);c3 = ([120 230 180]+128)/(256+128);patch([0 1 1 0],[0 0 1 1],c1);patch([0 1 1 1-e 1-e 0+e],[0 0 1 1-e 0+e 0+e],c2);patch([0 0 1 1-e 0+e 0+e],[0 1 1 1-e 1-e 0+e],c3);plot([0 1 1 0 0],[0 0 1 1 0]);
  y : Outport  PortDimensions=5
      mask Display: e = 0.06;c1 = [120 230 180]/255;c2 = ([120 230 180]+0)/(256+128);c3 = ([120 230 180]+128)/(256+128);patch([0 1 1 0],[0 0 1 1],c1);patch([0 1 1 1-e 1-e 0+e],[0 0 1 1-e 0+e 0+e],c2);patch([0 0 1 1-e 0+e 0+e],[0 1 1 1-e 1-e 0+e],c3);plot([0 1 1 0 0],[0 0 1 1 0]);
  wires:
  a/1 -> y/1

== system AcasXuClosedLoop_all_comp2017b/networks/Networks_m_2/AcasXu_4_2/Layer 1 ==
  "p{1}" : Inport  PortDimensions=5
      mask Display: e = 0.06;c1 = [120 230 180]/255;c2 = ([120 230 180]+0)/(256+128);c3 = ([120 230 180]+128)/(256+128);patch([0 1 1 0],[0 0 1 1],c1);patch([0 1 1 1-e 1-e 0+e],[0 0 1 1-e 0+e 0+e],c2);patch([0 0 1 1-e 0+e 0+e],[0 1 1 1-e 1-e 0+e],c3);plot([0 1 1 0 0],[0 0 1 1 0]);
  Delays 1 : Subsystem
      mask Display: e = 0.06;c1 = [120 150 230]/255;c2 = ([120 150 230]+0)/383;c3 = ([120 150 230]+128)/383;patch([0 1 1 0],[0 0 1 1],c1);patch([0 1 1 1-e 1-e 0+e],[0 0 1 1-e 0+e 0+e],c2);patch([0 0 1 1-e 0+e 0+e],[0 1 1 1-e 1-e 0+e],c3);plot([0 1 1 0 0],[0 0 1 1 0]);disp('0');
  "IW{1,1}" : Subsystem
      mask Display: e = 0.06;c1 = [120 150 230]/255;c2 = ([120 150 230]+0)/383;c3 = ([120 150 230]+128)/383;patch([0 1 1 0],[0 0 1 1],c1);patch([0 1 1 1-e 1-e 0+e],[0 0 1 1-e 0+e 0+e],c2);patch([0 0 1 1-e 0+e 0+e],[0 1 1 1-e 1-e 0+e],c3);plot([0 1 1 0 0],[0 0 1 1 0]);disp('W');
  "b{1}" : Constant  Value=[0.057281800000000000772093500245318864472210407257080078125;-0.126450000000000006838973831690964289009571075439453125;-0.223335000000000005737632591262809000909328460693359375;-0.045713099999999999567545927448009024374186992645263671875;-0.26653599999999999514699311475851573050022125244140625;0.08256239999999999412683138189095188863575458526611328125;0.18873200000000001086419842977193184196949005126953125;-0.472499999999999975575093458246556110680103302001953125;-0.197755999999999987348786589791416190564632415771484375;-0.368535000000000001474376176702207885682582855224609375;0.00194447999999999994978627793074110741144977509975433349609375;-0.140614999999999989999110994176589883863925933837890625;-0.1872689999999999910240688905105344019830226898193359375;-0.03697799999999999698285790827867458574473857879638671875;-0.65614300000000003176836571583407931029796600341796875;-0.0230185000000000007658318423864329815842211246490478515625;-0.65889900000000001245581415787455625832080841064453125;-0.1425470000000000070361494408643920905888080596923828125;0.01334399999999999995858868118148166104219853878021240234375;-0.394174999999999997601918266809661872684955596923828125;0.266068000000000026705748723543365485966205596923828125;-0.0999339999999999950563989159491029568016529083251953125;-0.10625099999999999822453133901944966055452823638916015625;0.08811549999999999938093964146901271305978298187255859375;-0.56314699999999995316812828605179674923419952392578125;-0.019576799999999998369570874956480110995471477508544921875;-0.0281636000000000004395150909886069712229073047637939453125;-0.07745359999999999744968448567306040786206722259521484375;-0.6952760000000000051301185521879233419895172119140625;0.039308400000000000285016454881770187057554721832275390625;-0.7322509999999999852349219509051181375980377197265625;-0.52523900000000001142552719102241098880767822265625;-0.4020580000000000264748223344213329255580902099609375;0.1484000000000000041300296516055823303759098052978515625;-0.388166999999999984272136543950182385742664337158203125;0.10464900000000000590549831258613266982138156890869140625;0.1874159999999999992592591979700955562293529510498046875;-0.408720000000000027728930263037909753620624542236328125;-0.485524000000000011123546528324368409812450408935546875;0.0658847999999999933695704612546251155436038970947265625;0.0198997999999999987397192313665073015727102756500244140625;-0.05769760000000000166320290873045451007783412933349609375;-0.418300999999999978395948119214153848588466644287109375;-0.2046769999999999978257392285740934312343597412109375;-0.42998300000000000409272615797817707061767578125;-0.1618380000000000096260777127099572680890560150146484375;-0.2171909999999999951736384673495194874703884124755859375;-0.0020542500000000000863475957402215499314479529857635498046875;-0.1920300000000000062438942904918803833425045013427734375;-0.273278999999999994141575143657973967492580413818359375]
      mask Display: e = 0.06;c1 = [120 150 230]/255;c2 = ([120 150 230]+0)/383;c3 = ([120 150 230]+128)/383;patch([0 1 1 0],[0 0 1 1],c1);patch([0 1 1 1-e 1-e 0+e],[0 0 1 1-e 0+e 0+e],c2);patch([0 0 1 1-e 0+e 0+e],[0 1 1 1-e 1-e 0+e],c3);plot([0 1 1 0 0],[0 0 1 1 0]);disp('b');
  netsum : Sum  Inputs=2  SaturateOnIntegerOverflow=on  InputSameDT=on  OutDataTypeStr=Inherit: Same as first input
      mask Display: e = 0.06;c1 = [120 150 230]/255;c2 = ([120 150 230]+0)/383;c3 = ([120 150 230]+128)/383;patch([0 1 1 0],[0 0 1 1],c1);patch([0 1 1 1-e 1-e 0+e],[0 0 1 1-e 0+e 0+e],c2);patch([0 0 1 1-e 0+e 0+e],[0 1 1 1-e 1-e 0+e],c3);plot([0 1 1 0 0],[0 0 1 1 0]);disp('+');
  poslin : neural/Transfer Functions/poslin
      settings: SourceProductName=Deep Learning Toolbox  SourceProductBaseCode=NN
  "a{1}" : Outport  InitialOutput=[0;0;0;0;0;0;0;0;0;0;0;0;0;0;0;0;0;0;0;0;0;0;0;0;0;0;0;0;0;0;0;0;0;0;0;0;0;0;0;0;0;0;0;0;0;0;0;0;0;0]
      mask Display: e = 0.06;c1 = [120 230 180]/255;c2 = ([120 230 180]+0)/(256+128);c3 = ([120 230 180]+128)/(256+128);patch([0 1 1 0],[0 0 1 1],c1);patch([0 1 1 1-e 1-e 0+e],[0 0 1 1-e 0+e 0+e],c2);patch([0 0 1 1-e 0+e 0+e],[0 1 1 1-e 1-e 0+e],c3);plot([0 1 1 0 0],[0 0 1 1 0]);
  wires:
  poslin/1 -> "a{1}"/1
  netsum/1 -> poslin/1
  "b{1}"/1 -> netsum/2
  "IW{1,1}"/1("iz{1,1}") -> netsum/1
  Delays 1/1("pd{1,1}") -> "IW{1,1}"/1("pd{1,1}")
  "p{1}"/1 -> Delays 1/1("p{1}")

== system AcasXuClosedLoop_all_comp2017b/networks/Networks_m_2/AcasXu_4_2/Layer 2 ==
  "a{1} " : Inport  PortDimensions=50
      mask Display: e = 0.06;c1 = [120 230 180]/255;c2 = ([120 230 180]+0)/(256+128);c3 = ([120 230 180]+128)/(256+128);patch([0 1 1 0],[0 0 1 1],c1);patch([0 1 1 1-e 1-e 0+e],[0 0 1 1-e 0+e 0+e],c2);patch([0 0 1 1-e 0+e 0+e],[0 1 1 1-e 1-e 0+e],c3);plot([0 1 1 0 0],[0 0 1 1 0]);
  Delays 1 : Subsystem
      mask Display: e = 0.06;c1 = [120 150 230]/255;c2 = ([120 150 230]+0)/383;c3 = ([120 150 230]+128)/383;patch([0 1 1 0],[0 0 1 1],c1);patch([0 1 1 1-e 1-e 0+e],[0 0 1 1-e 0+e 0+e],c2);patch([0 0 1 1-e 0+e 0+e],[0 1 1 1-e 1-e 0+e],c3);plot([0 1 1 0 0],[0 0 1 1 0]);disp('0');
  "LW{2,1}" : Subsystem
      mask Display: e = 0.06;c1 = [120 150 230]/255;c2 = ([120 150 230]+0)/383;c3 = ([120 150 230]+128)/383;patch([0 1 1 0],[0 0 1 1],c1);patch([0 1 1 1-e 1-e 0+e],[0 0 1 1-e 0+e 0+e],c2);patch([0 0 1 1-e 0+e 0+e],[0 1 1 1-e 1-e 0+e],c3);plot([0 1 1 0 0],[0 0 1 1 0]);disp('W');
  "b{2}" : Constant  Value=[0.09422419999999999407069850576590397395193576812744140625;-0.024317800000000000526512167198234237730503082275390625;-1.403350000000000097344354799133725464344024658203125;0.59581899999999998751576413269503973424434661865234375;-0.017708000000000001517008740847813896834850311279296875;-0.6723749999999999449329379785922355949878692626953125;-0.477067999999999992066790355238481424748897552490234375;-0.52623100000000000431299440606380812823772430419921875;-0.365736000000000005538680625249980948865413665771484375;-0.20972299999999999275956952260457910597324371337890625;-0.63612199999999996524735479397349990904331207275390625;0.0717032999999999975937470253484207205474376678466796875;-0.3569539999999999935198502498678863048553466796875;-0.67140999999999995129229546364513225853443145751953125;-0.68023599999999995180388623339240439236164093017578125;-0.63552299999999994906119127335841767489910125732421875;-0.11976299999999999446043119633031892590224742889404296875;0.562446000000000001506350599811412394046783447265625;0.67920499999999994766852751126862131059169769287109375;0.50624999999999997779553950749686919152736663818359375;-0.43035699999999998954791635696892626583576202392578125;-0.45877000000000001111999381464556790888309478759765625;0.04880389999999999728341748550519696436822414398193359375;-0.04835810000000000108411057908597285859286785125732421875;-0.7367749999999999577227072222740389406681060791015625;0.483731999999999995321076085019740276038646697998046875;0.392266999999999976811437818469130434095859527587890625;-0.923834999999999961772800816106610000133514404296875;-0.1526679999999999981508125301843392662703990936279296875;0.257045000000000023465673848477308638393878936767578125;0.52027900000000004698819111581542529165744781494140625;-0.76651199999999997114485950078233145177364349365234375;0.66032100000000004680345000451779924333095550537109375;0.74048199999999997356070480236667208373546600341796875;-0.92521799999999998487254515566746704280376434326171875;1.2142900000000000915179043659009039402008056640625;0.410225000000000006306066779870889149606227874755859375;-0.3205459999999999975983655531308613717555999755859375;0.477710000000000023501200985265313647687435150146484375;0.110655000000000003357314426466473378241062164306640625;-0.84866200000000002745537130977027118206024169921875;-0.006802840000000000102164943172056155162863433361053466796875;-0.1638219999999999954010121427927515469491481781005859375;-0.01054040000000000011637357744120890856720507144927978515625;-0.78840699999999996894217701992602087557315826416015625;-0.04823170000000000234852137737107113935053348541259765625;-0.7505159999999999609343603879096917808055877685546875;-0.99586399999999997145749830451677553355693817138671875;0.5134009999999999962483343551866710186004638671875;-0.0107124000000000003940403558999605593271553516387939453125]
      mask Display: e = 0.06;c1 = [120 150 230]/255;c2 = ([120 150 230]+0)/383;c3 = ([120 150 230]+128)/383;patch([0 1 1 0],[0 0 1 1],c1);patch([0 1 1 1-e 1-e 0+e],[0 0 1 1-e 0+e 0+e],c2);patch([0 0 1 1-e 0+e 0+e],[0 1 1 1-e 1-e 0+e],c3);plot([0 1 1 0 0],[0 0 1 1 0]);disp('b');
  netsum : Sum  Inputs=2  SaturateOnIntegerOverflow=on  InputSameDT=on  OutDataTypeStr=Inherit: Same as first input
      mask Display: e = 0.06;c1 = [120 150 230]/255;c2 = ([120 150 230]+0)/383;c3 = ([120 150 230]+128)/383;patch([0 1 1 0],[0 0 1 1],c1);patch([0 1 1 1-e 1-e 0+e],[0 0 1 1-e 0+e 0+e],c2);patch([0 0 1 1-e 0+e 0+e],[0 1 1 1-e 1-e 0+e],c3);plot([0 1 1 0 0],[0 0 1 1 0]);disp('+');
  poslin : neural/Transfer Functions/poslin
      settings: SourceProductName=Deep Learning Toolbox  SourceProductBaseCode=NN
  "a{2}" : Outport  InitialOutput=[0;0;0;0;0;0;0;0;0;0;0;0;0;0;0;0;0;0;0;0;0;0;0;0;0;0;0;0;0;0;0;0;0;0;0;0;0;0;0;0;0;0;0;0;0;0;0;0;0;0]
      mask Display: e = 0.06;c1 = [120 230 180]/255;c2 = ([120 230 180]+0)/(256+128);c3 = ([120 230 180]+128)/(256+128);patch([0 1 1 0],[0 0 1 1],c1);patch([0 1 1 1-e 1-e 0+e],[0 0 1 1-e 0+e 0+e],c2);patch([0 0 1 1-e 0+e 0+e],[0 1 1 1-e 1-e 0+e],c3);plot([0 1 1 0 0],[0 0 1 1 0]);
  wires:
  poslin/1 -> "a{2}"/1
  netsum/1 -> poslin/1
  "b{2}"/1 -> netsum/2
  "LW{2,1}"/1("lz{2,1}") -> netsum/1
  Delays 1/1("pd{2,1}") -> "LW{2,1}"/1("ad{2,1}")
  "a{1} "/1 -> Delays 1/1("p{2}")
